simulink model "BMSModel"  (R2024b)
settings: Solver=VariableStepAuto  StartTime=0.0  StopTime=+inf  RelTol=1e-3
undefined symbols (not defined in the file): balancingRelaxationTime, balancingTime, ChargerParRes, ChargerRes, ChargingCurrentRequest, DeltaVTargetMin, DrivingCurrentRqst, InverterParRes, InverterRes, OpenCircuitInjection, PassiveBalancingRes, ShortCircuitInjection, sigbuilder_block, SystemState, SystemState_t
libraries: fl_lib x325, nesl_utility x223, batteryecm_lib x30, ee_lib x30
(part 1 of 33)
settings Model: SimulationMode=accelerator  Creator=E14  LastModifiedBy=E14

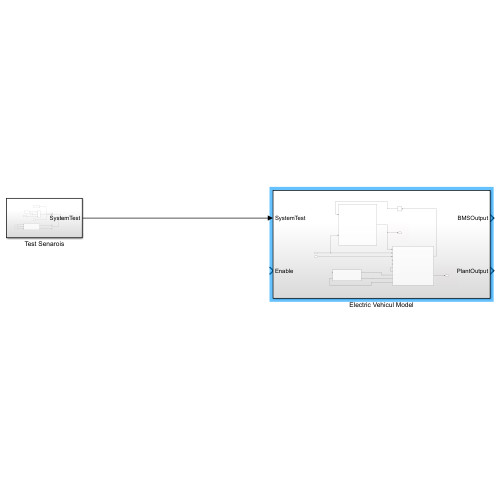
[thumbnail: Simulink's stored preview of the top-level diagram]
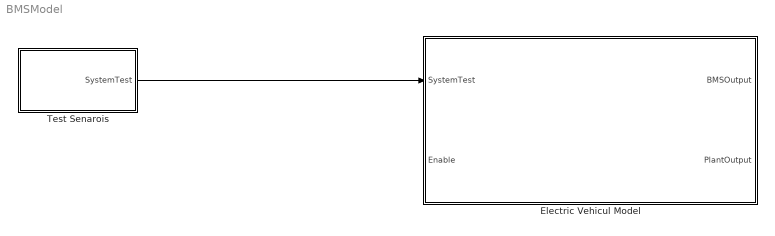
== system BMSModel ==
  Electric Vehicul Model : Subsystem
  Test Senarois : Subsystem
  wires:
  Test Senarois/1(SystemTest) -> Electric Vehicul Model/1(SystemTest)

== system BMSModel/Electric Vehicul Model ==
  SystemTest : Inport
  Enable : Inport  Port=2
  BMS model : Subsystem
  Contactors : Subsystem
  HV Battery : Subsystem
  Unit Delay : UnitDelay  InitialCondition=0.01  SampleTime=-1  HasFrameUpgradeWarning=on
  BMSOutput : Outport  OutDataTypeStr=Bus: BusOutputBMS
  PlantOutput : Outport  Port=2  OutDataTypeStr=Bus: BusSimulationOutput
  wires:
  BMS model/1(ToHVBattery) -> BMSOutput/1, HV Battery/1(DataFromBMS)
  Unit Delay/1 -> BMS model/1(DataFromHVBattery)
  HV Battery/1(ToBMS) -> Unit Delay/1
  SystemTest/1 -> HV Battery/2(SimulationTest), BMS model/2(SimulationTest)
  HV Battery/2(SimulationOutput) -> PlantOutput/1
  Enable/1 -> HV Battery/3(Enable)
  connections:
  HV Battery/LConn1 -- HV Battery/LConn2
  HV Battery/LConn5 -- Contactors/LConn1
  HV Battery/LConn3 -- Contactors/RConn1
  HV Battery/LConn4 -- Contactors/RConn2

== system BMSModel/Test Senarois ==
  Bus Creator : BusCreator  Inputs=SystemState,DrivingCurrentRqst,ChargingCurrentRequest  OutDataTypeStr=Bus: SimulationSystemInput
  Bus Creator1 : BusCreator  Inputs=ShortCircuitInjection,OpenCircuitInjection  OutDataTypeStr=Bus: SimulationBatteryPackInput
  Bus Creator2 : BusCreator  Inputs=2  OutDataTypeStr=Bus: BusSimulationInput  NonVirtualBus=on
  ChargingCurrentRqst : Constant  Value=42.56966044823757
  DrivingCurrentRqst : Constant  Value=30.1857592269321
  DrivingCurrentSelector : Constant
  MATLAB Function : MATLAB Function  TreatAsAtomicUnit=on
  ModelNumber : Constant  Value=3
  Signal Builder : Subsystem  Tag=STV Subsys  LoadFcn=sigbuilder_block('load');  StartFcn=sigbuilder_block('start');  StopFcn=sigbuilder_block('stop');  ClipboardFcn=sigbuilder_block('clipboard');  mask(Sigbuilder block){tuvar=[0:10]}
      mask help: Simulink:masks:SigbuilderBlock_MD
      mask init:
      | if ~strcmp(get_param(bdroot(gcbh),'SimulationStatus'),'stopped') tuvar = sigbuilder_block('maskInit'); end
      settings: CopyFcn=sigbuilder_block('copy');  DeleteFcn=sigbuilder_block('delete');  ModelCloseFcn=sigbuilder_block('modelClose');  PreSaveFcn=sigbuilder_block('preSave');  PauseFcn=sigbuilder_block('pause');  ContinueFcn=sigbuilder_block('continue');  NameChangeFcn=sigbuilder_block('namechange');  OpenFcn=sigbuilder_block('open',[5.4 5.4 1141.2 580.8 ]);  CloseFcn=sigbuilder_block('close');
      mask Display: plot(0, 0, 100, 100,[2, 2, 32, 32, 2], [68, 8, 8, 68, 68],[32, 2], [38, 38], [32, 19, 2],[53, 60, 44], [32, 17, 17, 2],[16, 16, 31, 31]);txt = getActiveGroup(gcbh);text(2, 100, txt,'verticalAlignment', 'top');
  Switch : Switch  Threshold=2  SaturateOnIntegerOverflow=off  InputSameDT=off
  SystemState : Constant  Value=SystemState_t(1)  OutDataTypeStr=Enum: SystemState_t
  cellNumber : Constant  Value=2
  faultType : Constant  Value=0
  SystemTest : Outport  OutDataTypeStr=Bus: BusSimulationInput
  wires:
  faultType/1 -> MATLAB Function/1(faultType)
  ChargingCurrentRqst/1 -> Bus Creator/3  "ChargingCurrentRequest"
  SystemState/1 -> Bus Creator/1  "SystemState"
  Bus Creator1/1 -> Bus Creator2/2  "SimulationBatteryPackInput"
  DrivingCurrentSelector/1 -> Switch/2
  Signal Builder/1(Current) -> Switch/1
  MATLAB Function/1(SCInjVector) -> Bus Creator1/1  "SCInjection"
  DrivingCurrentRqst/1 -> Switch/3
  Switch/1 -> Bus Creator/2  "DrivingCurrentRqst"
  MATLAB Function/2(OCInjVector) -> Bus Creator1/2  "OCInjection"
  Bus Creator/1 -> Bus Creator2/1  "SimulationSystemInput"
  cellNumber/1 -> MATLAB Function/3(cellNumber)
  ModelNumber/1 -> MATLAB Function/2(ModelNumber)
  Bus Creator2/1 -> SystemTest/1  "BusSimulationInput"

== system BMSModel/Electric Vehicul Model/BMS model ==
  DataFromHVBattery : Inport
  SimulationTest : Inport  Port=2
  Balancing system : Subsystem
  Bus Creator : BusCreator  Inputs=3
  Bus Selector : BusSelector  OutputSignals=BatteryPackData.ModelVoltageVector
  Bus Selector1 : BusSelector  OutputSignals=signal1
  Bus Selector2 : BusSelector  OutputSignals=BatteryPackData.ModelCurrentVector,BatteryPackData.ModelVoltageVector,BatteryPackData.ModelTempVector
  SOC_Estimation : Subsystem
  ToHVBattery : Outport
  wires:
  DataFromHVBattery/1 -> Bus Selector2/1, Bus Selector/1
  Bus Selector/1 -> Balancing system/2(SegmentVoltageVector)  "<ModelVoltageVector>"
  SimulationTest/1 -> Bus Selector1/1
  Bus Selector1/1 -> Balancing system/1(SystemState)  "<signal1>"
  Balancing system/1(balancingCmd) -> Bus Creator/1  "balancingCmd"
  Balancing system/2(balancingDeltaVCell) -> Bus Creator/2  "balancingDeltaVCell"
  Balancing system/3(balancingFlags) -> Bus Creator/3  "balancingFlags"
  Bus Creator/1 -> ToHVBattery/1  "Balancing"
  Bus Selector2/1 -> SOC_Estimation/1(In2)  "<ModelCurrentVector>"
  Bus Selector2/2 -> SOC_Estimation/2(In1)  "<ModelVoltageVector>"
  Bus Selector2/3 -> SOC_Estimation/3(In3)  "<ModelTempVector>"

== system BMSModel/Electric Vehicul Model/Contactors ==
  DichargerCmd : Inport
  Bat+ : PMIOPort  Side=Left
  Charger : PMIOPort  Port=2  Side=Right
  Inverter+ : PMIOPort  Port=3  Side=Right

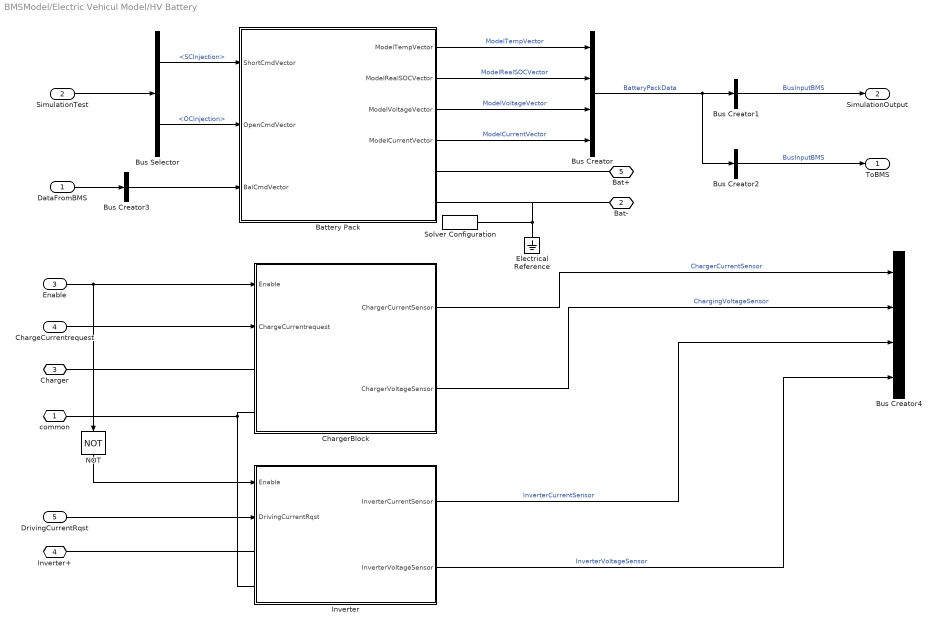
== system BMSModel/Electric Vehicul Model/HV Battery ==
  DataFromBMS : Inport
  SimulationTest : Inport  Port=2  OutDataTypeStr=Bus: BusSimulationInput
  Enable : Inport  Port=3
  ChargeCurrentrequest : Inport  Port=4
  DrivingCurrentRqst : Inport  Port=5
  Battery Pack : Subsystem
  Bus Creator : BusCreator  Inputs=4
  Bus Creator1 : BusCreator  Inputs=1
  Bus Creator2 : BusCreator  Inputs=1
  Bus Creator3 : BusCreator  Inputs=1
  Bus Creator4 : BusCreator  Inputs=4
  Bus Selector : BusSelector  OutputSignals=SimulationBatteryPackInput.SCInjection,SimulationBatteryPackInput.OCInjection
  ChargerBlock : Subsystem
  Electrical Reference : fl_lib/Electrical/Electrical Elements/Electrical Reference
  Inverter : Subsystem
  NOT : Logic  Operator=NOT  IconShape=distinctive  AllPortsSameDT=off  OutDataTypeStr=boolean
  Solver Configuration : nesl_utility/Solver Configuration  PhysicalDomain=network_engine_domain  SubClassName=solver  LeftPortType=input  RightPortType=generic  Tones=[]  Harmonics=[]  SimulateNoise=off  Profile=off  UseLocalSampling=off  EnableSwitchedLinearOptims=on  FrequencyDomain=off  UseCCode=off  RelTol=0.001  AbsTol=1e-06  MinStep=1e-09  ToWorkspace=off  RevertToSquareIcSolve=off  NumHomotopyIterations=100  PhasorMode=off  PhasorModeNumHarmonics=1  ConsistencySolver=NEWTON_FTOL  IndexReductionMethod=DerivativeReplacement  DoDC=off  ResidualTolerance=1e-09  ConsistencyTolSource=GLOBAL  ConsistencyAbsTol=0.001  ConsistencyRelTol=0.001  ConsistencyTolFactor=0.001  UseLocalSolver=off  LocalSolverChoice=NE_BACKWARD_EULER_ADVANCER  ResolveIndetEquations=on  FunctionEvalNumThread=1  LocalSolverSampleTime=0.001  DoFixedCost=off  MaxNonlinIter=3  MaxModeIter=2  ComputeImpulses=off  ImpulseIterations=2  LinearAlgebra=auto  LinearAlgebraNumThread=0  EquationFormulation=NE_TIME_EF  DelaysMemoryBudget=1024  AutomaticFiltering=on  FilteringTimeConstant=0.001  PartitionStorageMethod=AS_NEEDED  PartitionMemoryBudget=1024  PartitionMethod=ROBUST  MultibodyUseLocalSolver=off  MultibodyLocalSolverChoice=ODE1  MultibodyLocalSolverSampleTime=0.001
  common : PMIOPort  Side=Left
  Bat- : PMIOPort  Port=2  Side=Left
  Charger : PMIOPort  Port=3  Side=Left
  Inverter+ : PMIOPort  Port=4  Side=Left
  Bat+ : PMIOPort  Port=5  Side=Left
  ToBMS : Outport  OutDataTypeStr=Bus: BusSimulationOutput
  SimulationOutput : Outport  Port=2  OutDataTypeStr=Bus: BusSimulationOutput
  wires:
  Battery Pack/2(ModelRealSOCVector) -> Bus Creator/2  "ModelRealSOCVector"
  Bus Creator/1 -> Bus Creator2/1, Bus Creator1/1  "BatteryPackData"
  Battery Pack/1(ModelTempVector) -> Bus Creator/1  "ModelTempVector"
  Bus Creator1/1 -> SimulationOutput/1  "BusInputBMS"
  Bus Selector/2 -> Battery Pack/2(OpenCmdVector)  "<OCInjection>"
  Battery Pack/4(ModelCurrentVector) -> Bus Creator/4  "ModelCurrentVector"
  Bus Selector/1 -> Battery Pack/1(ShortCmdVector)  "<SCInjection>"
  Battery Pack/3(ModelVoltageVector) -> Bus Creator/3  "ModelVoltageVector"
  Bus Creator2/1 -> ToBMS/1  "BusInputBMS"
  SimulationTest/1 -> Bus Selector/1
  DataFromBMS/1 -> Bus Creator3/1
  Bus Creator3/1 -> Battery Pack/3(BalCmdVector)
  ChargerBlock/1(ChargerCurrentSensor) -> Bus Creator4/1  "ChargerCurrentSensor"
  ChargerBlock/2(ChargerVoltageSensor) -> Bus Creator4/2  "ChargingVoltageSensor"
  Inverter/1(InverterCurrentSensor) -> Bus Creator4/3  "InverterCurrentSensor"
  Inverter/2(InverterVoltageSensor) -> Bus Creator4/4  "InverterVoltageSensor"
  Enable/1 -> ChargerBlock/1(Enable), NOT/1
  NOT/1 -> Inverter/1(Enable)
  ChargeCurrentrequest/1 -> ChargerBlock/2(ChargeCurrentrequest)
  DrivingCurrentRqst/1 -> Inverter/2(DrivingCurrentRqst)
  connections:
  Solver Configuration/RConn1 -- Battery Pack/RConn2 -- Bat-/RConn1 -- Electrical Reference/LConn1
  Inverter/LConn2 -- common/RConn1 -- ChargerBlock/LConn2
  Charger/RConn1 -- ChargerBlock/LConn1
  Inverter+/RConn1 -- Inverter/LConn1
  Bat+/RConn1 -- Battery Pack/RConn1

== system BMSModel/Test Senarois/Signal Builder ==
  Demux : Demux  Tag=STV Demux  Outputs=1
      settings: DisplayOption=none
  FromWs : FromWorkspace  Tag=STV FromWs  VariableName=tuvar
      SigBuilderData=|
      | common.afterFinalStr = 'Extrapolate'
      | common.sampleTime = 0
      | dataSet.timeRange = [0 2474]
      | dataSet.name = 'FTP-75 Drive Cycle'
      | sbobj.Groups.Name = 'FTP-75 Drive Cycle'
      | sbobj.Groups.Signals.Name = 'Current'
      | sbobj.Groups.Signals.XData = [0 0.00166966283167 0.00489865696245 0.00887290141926 0.01 0.0132228027433 0.0164456054866 0.0196684082299 0.02 0.0216579588505 0.0216579588505 0.023878217756 ... (264079 elements, 1x264079)]
      | sbobj.Groups.Signals.YData = [0 0 0 0 0 0 0 0 0 0 -7.47487403974e-31 -1.06202348499e-17 ... (264079 elements, 1x264079)]
      | sbobj.Groups.TimeRange = [0 2474]
  Current : Outport  Tag=STV Outport
  wires:
  FromWs/1 -> Demux/1
  Demux/1 -> Current/1

== system BMSModel/Electric Vehicul Model/BMS model/Balancing system ==
  SystemState : Inport  OutDataTypeStr=Enum: SystemState_t
  SegmentVoltageVector : Inport  Port=2
  Chart1 : Stateflow chart  TreatAsAtomicUnit=on
      settings: PortSchema="{\"entries\":[{\"content\":{\"sides\":[{\"content\":{\"connectorIds\":[\"In1\",\"In2\",\"In3\",\"In4\"]},\"type\":\"ConnectorPlacement.EquallySpacedRectSide\",\"uuid\":\"a2741edf-0a6a-4cdc-8864-68b68dbb5e7b\"},{\"content\":{\"connectorIds\":[\"Out2\",\"Out1\",\"Out3\"],\"side\":\"RIGHT\"},\"type\":\"ConnectorPlacement.EquallySpacedRectSide\",\"uuid\":\"3e33edf4-8d09-490e-8b71-f55d7866ff69\"},{\"content\":{\"connectorIds\":[],\"side\":\"TOP\"},\"type\":\"ConnectorPlacement.EquallySpacedRectSide\",\"uuid\":\"52eb0d7a-a4df-4df7-91e4-464aa4e0ab31\"}]},\"type\":\"ConnectorPlacement.EquallySpacedConnector\",\"uuid\":\"8927709b-3b25-412c-93a6-8f62d2d50716\"}],\"packageUris\":[\"http://schema.mathworks.com/mf0/Connector/R2020b\"],\"version\":\"1.0\"}"
  Chart2 : Stateflow chart  TreatAsAtomicUnit=on
      settings: PortSchema="{\"entries\":[{\"content\":{\"sides\":[{\"content\":{\"connectorIds\":[\"In1\",\"In2\",\"In3\",\"In4\"]},\"type\":\"ConnectorPlacement.EquallySpacedRectSide\",\"uuid\":\"a2741edf-0a6a-4cdc-8864-68b68dbb5e7b\"},{\"content\":{\"connectorIds\":[\"Out2\",\"Out1\",\"Out3\"],\"side\":\"RIGHT\"},\"type\":\"ConnectorPlacement.EquallySpacedRectSide\",\"uuid\":\"3e33edf4-8d09-490e-8b71-f55d7866ff69\"},{\"content\":{\"connectorIds\":[],\"side\":\"TOP\"},\"type\":\"ConnectorPlacement.EquallySpacedRectSide\",\"uuid\":\"52eb0d7a-a4df-4df7-91e4-464aa4e0ab31\"}]},\"type\":\"ConnectorPlacement.EquallySpacedConnector\",\"uuid\":\"8927709b-3b25-412c-93a6-8f62d2d50716\"}],\"packageUris\":[\"http://schema.mathworks.com/mf0/Connector/R2020b\"],\"version\":\"1.0\"}"
  Chart3 : Stateflow chart  TreatAsAtomicUnit=on
      settings: PortSchema="{\"entries\":[{\"content\":{\"sides\":[{\"content\":{\"connectorIds\":[\"In1\",\"In2\",\"In3\",\"In4\"]},\"type\":\"ConnectorPlacement.EquallySpacedRectSide\",\"uuid\":\"a2741edf-0a6a-4cdc-8864-68b68dbb5e7b\"},{\"content\":{\"connectorIds\":[\"Out2\",\"Out1\",\"Out3\"],\"side\":\"RIGHT\"},\"type\":\"ConnectorPlacement.EquallySpacedRectSide\",\"uuid\":\"3e33edf4-8d09-490e-8b71-f55d7866ff69\"},{\"content\":{\"connectorIds\":[],\"side\":\"TOP\"},\"type\":\"ConnectorPlacement.EquallySpacedRectSide\",\"uuid\":\"52eb0d7a-a4df-4df7-91e4-464aa4e0ab31\"}]},\"type\":\"ConnectorPlacement.EquallySpacedConnector\",\"uuid\":\"8927709b-3b25-412c-93a6-8f62d2d50716\"}],\"packageUris\":[\"http://schema.mathworks.com/mf0/Connector/R2020b\"],\"version\":\"1.0\"}"
  Matrix Concatenate : Concatenate  NumInputs=3  Mode=Multidimensional array  ConcatenateDimension=2
  Max : MinMax  Function=max
  Max1 : MinMax  Function=max
  Max2 : MinMax  Function=max
  Min : MinMax
  Min1 : MinMax
  Min2 : MinMax
  Min3 : MinMax  Function=max  Inputs=3
  Min4 : MinMax  Inputs=3
  Selector : Selector  NumberOfDimensions=2  InputPortWidth=3  IndexOptions=Starting index (dialog),Select all  Indices=1,1  OutputSizes=1,1
  Selector1 : Selector  NumberOfDimensions=2  InputPortWidth=3  IndexOptions=Starting index (dialog),Select all  Indices=2,1  OutputSizes=1,1
  Selector2 : Selector  NumberOfDimensions=2  InputPortWidth=3  IndexOptions=Starting index (dialog),Select all  Indices=3,1  OutputSizes=1,1
  Transpose : Math  Operator=transpose
  Vector Concatenate : Concatenate  NumInputs=3
  Vector Concatenate1 : Concatenate  NumInputs=3
  balancingCmd : Outport
  balancingDeltaVCell : Outport  Port=2
  balancingFlags : Outport  Port=3
  wires:
  Selector2/1 -> Max2/1, Min2/1, Chart3/2(SegmentVoltageVector)
  Transpose/1 -> balancingCmd/1
  Selector/1 -> Max/1, Min/1, Chart1/2(SegmentVoltageVector)
  Min3/1 -> Chart2/3(CellMaxV), Chart1/3(CellMaxV), Chart3/3(CellMaxV)
  Min/1 -> Min4/1
  SystemState/1 -> Chart2/1(SystemState), Chart3/1(SystemState), Chart1/1(SystemState)
  Min4/1 -> Chart2/4(CellMinV), Chart1/4(CellMinV), Chart3/4(CellMinV)
  SegmentVoltageVector/1 -> Selector/1, Selector1/1, Selector2/1
  Chart1/2(DeltaVCell) -> Vector Concatenate/1
  Min2/1 -> Min4/3
  Chart1/1(balancingCmd) -> Matrix Concatenate/1
  Chart1/3(balancingFlag) -> Vector Concatenate1/1
  Selector1/1 -> Min1/1, Max1/1, Chart2/2(SegmentVoltageVector)
  Matrix Concatenate/1 -> Transpose/1
  Chart3/1(balancingCmd) -> Matrix Concatenate/3
  Max2/1 -> Min3/3
  Chart2/1(balancingCmd) -> Matrix Concatenate/2
  Chart2/2(DeltaVCell) -> Vector Concatenate/2
  Max/1 -> Min3/1
  Chart3/2(DeltaVCell) -> Vector Concatenate/3
  Chart2/3(balancingFlag) -> Vector Concatenate1/2
  Chart3/3(balancingFlag) -> Vector Concatenate1/3
  Vector Concatenate1/1 -> balancingFlags/1
  Min1/1 -> Min4/2
  Vector Concatenate/1 -> balancingDeltaVCell/1
  Max1/1 -> Min3/2

== system BMSModel/Electric Vehicul Model/BMS model/SOC_Estimation ==
  In2 : Inport
  In1 : Inport  Port=2
  In3 : Inport  Port=3
  Out1 : Outport

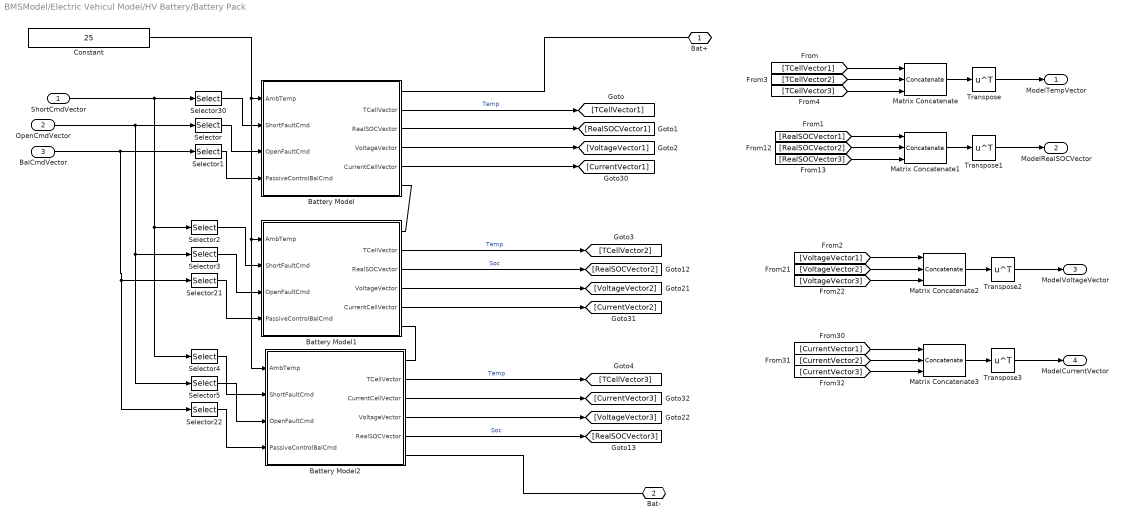
== system BMSModel/Electric Vehicul Model/HV Battery/Battery Pack ==
  ShortCmdVector : Inport
  OpenCmdVector : Inport  Port=2
  BalCmdVector : Inport  Port=3
  Battery Model : Subsystem
      settings: PortSchema="{\"entries\":[{\"content\":{\"sides\":[{\"content\":{\"connectorIds\":[\"In1\",\"In2\",\"In3\",\"In4\"]},\"type\":\"ConnectorPlacement.EquallySpacedRectSide\",\"uuid\":\"339fc2dc-73de-410a-a97e-1fe159b417b4\"},{\"content\":{\"connectorIds\":[\"RConn1\",\"Out1\",\"Out2\",\"Out3\",\"Out4\",\"RConn2\"],\"side\":\"RIGHT\"},\"type\":\"ConnectorPlacement.EquallySpacedRectSide\",\"uuid\":\"1ff17677-7e7c-48aa-9b47-364d19db4b22\"},{\"content\":{\"side\":\"TOP\"},\"type\":\"ConnectorPlacement.EquallySpacedRectSide\",\"uuid\":\"a6a1d56b-2099-4034-99b1-512debfe3694\"}]},\"type\":\"ConnectorPlacement.EquallySpacedConnector\",\"uuid\":\"fb04511b-02af-41ab-983c-5fc3ec8a5a5f\"}],\"packageUris\":[\"http://schema.mathworks.com/mf0/Connector/R2020b\"],\"version\":\"1.0\"}"
  Battery Model1 : Subsystem
      settings: PortSchema="{\"entries\":[{\"content\":{\"sides\":[{\"content\":{\"connectorIds\":[\"In1\",\"In2\",\"In3\",\"In4\"]},\"type\":\"ConnectorPlacement.EquallySpacedRectSide\",\"uuid\":\"339fc2dc-73de-410a-a97e-1fe159b417b4\"},{\"content\":{\"connectorIds\":[\"RConn1\",\"Out1\",\"Out2\",\"Out3\",\"Out4\",\"RConn2\"],\"side\":\"RIGHT\"},\"type\":\"ConnectorPlacement.EquallySpacedRectSide\",\"uuid\":\"1ff17677-7e7c-48aa-9b47-364d19db4b22\"},{\"content\":{\"side\":\"TOP\"},\"type\":\"ConnectorPlacement.EquallySpacedRectSide\",\"uuid\":\"a6a1d56b-2099-4034-99b1-512debfe3694\"}]},\"type\":\"ConnectorPlacement.EquallySpacedConnector\",\"uuid\":\"fb04511b-02af-41ab-983c-5fc3ec8a5a5f\"}],\"packageUris\":[\"http://schema.mathworks.com/mf0/Connector/R2020b\"],\"version\":\"1.0\"}"
  Battery Model2 : Subsystem
      settings: PortSchema="{\"entries\":[{\"content\":{\"sides\":[{\"content\":{\"connectorIds\":[\"In1\",\"In2\",\"In3\",\"In4\"]},\"type\":\"ConnectorPlacement.EquallySpacedRectSide\",\"uuid\":\"339fc2dc-73de-410a-a97e-1fe159b417b4\"},{\"content\":{\"connectorIds\":[\"RConn1\",\"Out1\",\"Out2\",\"Out3\",\"Out4\",\"RConn2\"],\"side\":\"RIGHT\"},\"type\":\"ConnectorPlacement.EquallySpacedRectSide\",\"uuid\":\"1ff17677-7e7c-48aa-9b47-364d19db4b22\"},{\"content\":{\"side\":\"TOP\"},\"type\":\"ConnectorPlacement.EquallySpacedRectSide\",\"uuid\":\"a6a1d56b-2099-4034-99b1-512debfe3694\"}]},\"type\":\"ConnectorPlacement.EquallySpacedConnector\",\"uuid\":\"fb04511b-02af-41ab-983c-5fc3ec8a5a5f\"}],\"packageUris\":[\"http://schema.mathworks.com/mf0/Connector/R2020b\"],\"version\":\"1.0\"}"
  Constant : Constant  Value=25
  Matrix Concatenate{,1..3} (4 alike) : Concatenate  NumInputs=3  Mode=Multidimensional array  ConcatenateDimension=2
  Selector : Selector  NumberOfDimensions=2  InputPortWidth=10  IndexOptions=Starting index (dialog),Select all  Indices=1,1  OutputSizes=1,1
  Selector1 : Selector  NumberOfDimensions=2  InputPortWidth=10  IndexOptions=Starting index (dialog),Select all  Indices=1,1  OutputSizes=1,1
  Selector2 : Selector  NumberOfDimensions=2  InputPortWidth=10  IndexOptions=Starting index (dialog),Select all  Indices=2,1  OutputSizes=1,1
  Selector21 : Selector  NumberOfDimensions=2  InputPortWidth=10  IndexOptions=Starting index (dialog),Select all  Indices=2,1  OutputSizes=1,1
  Selector22 : Selector  NumberOfDimensions=2  InputPortWidth=10  IndexOptions=Starting index (dialog),Select all  Indices=3,1  OutputSizes=1,1
  Selector3 : Selector  NumberOfDimensions=2  InputPortWidth=10  IndexOptions=Starting index (dialog),Select all  Indices=2,1  OutputSizes=1,1
  Selector30 : Selector  NumberOfDimensions=2  InputPortWidth=10  IndexOptions=Starting index (dialog),Select all  Indices=1,1  OutputSizes=1,1
  Selector4 : Selector  NumberOfDimensions=2  InputPortWidth=10  IndexOptions=Starting index (dialog),Select all  Indices=3,1  OutputSizes=1,1
  Selector5 : Selector  NumberOfDimensions=2  InputPortWidth=10  IndexOptions=Starting index (dialog),Select all  Indices=3,1  OutputSizes=1,1
  Transpose{,1..3} (4 alike) : Math  Operator=transpose
  Bat+ : PMIOPort  Side=Right
  Bat- : PMIOPort  Port=2  Side=Right
  ModelTempVector : Outport
  ModelRealSOCVector : Outport  Port=2
  ModelVoltageVector : Outport  Port=3
  ModelCurrentVector : Outport  Port=4
  wires:
  ShortCmdVector/1 -> Selector4/1, Selector2/1, Selector30/1
  Battery Model/1(TCellVector) -> [TCellVector1]  "Temp"
  Selector21/1 -> Battery Model1/4(PassiveControlBalCmd)
  OpenCmdVector/1 -> Selector5/1, Selector3/1, Selector/1
  Battery Model1/1(TCellVector) -> [TCellVector2]  "Temp"
  BalCmdVector/1 -> Selector22/1, Selector21/1, Selector1/1
  Battery Model1/3(VoltageVector) -> [VoltageVector2]
  Selector2/1 -> Battery Model1/2(ShortFaultCmd)
  Transpose2/1 -> ModelVoltageVector/1
  Matrix Concatenate1/1 -> Transpose1/1
  Matrix Concatenate3/1 -> Transpose3/1
  Battery Model2/2(CurrentCellVector) -> [CurrentVector3]
  Battery Model2/3(VoltageVector) -> [VoltageVector3]
  [TCellVector3] -> Matrix Concatenate/3
  [VoltageVector2] -> Matrix Concatenate2/2
  [TCellVector1] -> Matrix Concatenate/1
  Transpose3/1 -> ModelCurrentVector/1
  Constant/1 -> Battery Model2/1(AmbTemp), Battery Model1/1(AmbTemp), Battery Model/1(AmbTemp)
  Selector30/1 -> Battery Model/2(ShortFaultCmd)
  Battery Model2/1(TCellVector) -> [TCellVector3]  "Temp"
  [CurrentVector2] -> Matrix Concatenate3/2
  Selector3/1 -> Battery Model1/3(OpenFaultCmd)
  [RealSOCVector2] -> Matrix Concatenate1/2
  [CurrentVector3] -> Matrix Concatenate3/3
  Transpose1/1 -> ModelRealSOCVector/1
  [CurrentVector1] -> Matrix Concatenate3/1
  Matrix Concatenate2/1 -> Transpose2/1
  Transpose/1 -> ModelTempVector/1
  [RealSOCVector1] -> Matrix Concatenate1/1
  Matrix Concatenate/1 -> Transpose/1
  Selector5/1 -> Battery Model2/3(OpenFaultCmd)
  Selector/1 -> Battery Model/3(OpenFaultCmd)
  [RealSOCVector3] -> Matrix Concatenate1/3
  [TCellVector2] -> Matrix Concatenate/2
  Battery Model2/4(RealSOCVector) -> [RealSOCVector3]  "Soc"
  Battery Model1/2(RealSOCVector) -> [RealSOCVector2]  "Soc"
  [VoltageVector1] -> Matrix Concatenate2/1
  [VoltageVector3] -> Matrix Concatenate2/3
  Battery Model/2(RealSOCVector) -> [RealSOCVector1]
  Selector22/1 -> Battery Model2/4(PassiveControlBalCmd)
  Selector1/1 -> Battery Model/4(PassiveControlBalCmd)
  Battery Model/3(VoltageVector) -> [VoltageVector1]
  Battery Model/4(CurrentCellVector) -> [CurrentVector1]
  Selector4/1 -> Battery Model2/2(ShortFaultCmd)
  Battery Model1/4(CurrentCellVector) -> [CurrentVector2]
  connections:
  Battery Model1/RConn2 -- Battery Model2/RConn1
  Battery Model/RConn2 -- Battery Model1/RConn1
  Battery Model2/RConn2 -- Bat-/RConn1
  Battery Model/RConn1 -- Bat+/RConn1

== system BMSModel/Electric Vehicul Model/HV Battery/ChargerBlock ==
  Enable : Inport
  ChargeCurrentrequest : Inport  Port=2
  Cast To Double : DataTypeConversion  OutDataTypeStr=double  SaturateOnIntegerOverflow=off  RndMeth=Floor
  Cast To Double1 : DataTypeConversion  OutDataTypeStr=double  SaturateOnIntegerOverflow=off  RndMeth=Floor
  Cast To Double2 : DataTypeConversion  OutDataTypeStr=double  SaturateOnIntegerOverflow=off  RndMeth=Floor
  ChargerCap : fl_lib/Electrical/Electrical Elements/Capacitor  c=ChargerCap F  r=1e-6 Ohm  g=0 1/Ohm  i_specify=off  i_priority=None  i=0 A  i_nominal_specify=off  i_nominal_value=1  i_nominal_unit=A  v_specify=off  v_priority=None  v=0 V  v_nominal_specify=off  v_nominal_value=1  v_nominal_unit=V  vc_specify=off  vc_priority=High  vc=0 V  vc_nominal_specify=off  vc_nominal_value=1  vc_nominal_unit=V
  ChargerParResistance : fl_lib/Electrical/Electrical Elements/Resistor  R=ChargerParRes Ohm  i_specify=off  i_priority=None  i=0 A  i_nominal_specify=off  i_nominal_value=1  i_nominal_unit=A  v_specify=off  v_priority=None  v=0 V  v_nominal_specify=off  v_nominal_value=1  v_nominal_unit=V
  ChargerResistance : fl_lib/Electrical/Electrical Elements/Resistor  R=ChargerRes Ohm  i_specify=off  i_priority=None  i=0 A  i_nominal_specify=off  i_nominal_value=1  i_nominal_unit=A  v_specify=off  v_priority=None  v=0 V  v_nominal_specify=off  v_nominal_value=1  v_nominal_unit=V
  Constant3 : Constant  Value=0
  Controlled Current Source1 : fl_lib/Electrical/Electrical Sources/Controlled Current Source
  Current Sensor1 : fl_lib/Electrical/Electrical Sensors/Current Sensor
  PS-Simulink Converter : nesl_utility/PS-Simulink Converter  PhysicalDomain=network_engine_domain  SubClassName=ps_output  LeftPortType=input  RightPortType=output  PseudoPeriodic=off  Frequencies=[]  VectorFormat=1-D array  Unit=inherit  AffineConversion=off
  PS-Simulink Converter1 : nesl_utility/PS-Simulink Converter  PhysicalDomain=network_engine_domain  SubClassName=ps_output  LeftPortType=input  RightPortType=output  PseudoPeriodic=off  Frequencies=[]  VectorFormat=1-D array  Unit=inherit  AffineConversion=off
  Simulink-PS Converter1 : nesl_utility/Simulink-PS Converter  PhysicalDomain=network_engine_domain  SubClassName=ps_input  LeftPortType=input  RightPortType=output  PseudoPeriodic=off  Frequencies=[]  NoiseDistribution=none  NoiseParameters=[]  Unit=1  AffineConversion=off  FilteringAndDerivatives=provide  SimscapeFilterOrder=1  InputFilterTimeConstant=0.001  UdotUserProvided=0
  Switch1 : Switch  Criteria=u2 > Threshold  SaturateOnIntegerOverflow=off  InputSameDT=off
  Voltage Sensor1 : fl_lib/Electrical/Electrical Sensors/Voltage Sensor
  Charger+ : PMIOPort  Side=Left
  Charger- : PMIOPort  Port=2  Side=Left
  ChargerCurrentSensor : Outport
  ChargerVoltageSensor : Outport  Port=2
  wires:
  Cast To Double/1 -> Simulink-PS Converter1/1
  Constant3/1 -> Switch1/3
  PS-Simulink Converter/1 -> Cast To Double1/1
  PS-Simulink Converter1/1 -> Cast To Double2/1
  Cast To Double1/1 -> ChargerCurrentSensor/1
  Cast To Double2/1 -> ChargerVoltageSensor/1
  Enable/1 -> Switch1/2
  ChargeCurrentrequest/1 -> Switch1/1
  Switch1/1 -> Cast To Double/1
  connections:
  Controlled Current Source1/LConn1 -- Current Sensor1/LConn1
  Voltage Sensor1/LConn1 -- ChargerResistance/RConn1 -- Current Sensor1/RConn2
  Simulink-PS Converter1/RConn1 -- Controlled Current Source1/RConn1
  ChargerCap/LConn1 -- Charger+/RConn1 -- ChargerParResistance/LConn1 -- ChargerResistance/LConn1
  PS-Simulink Converter1/LConn1 -- Voltage Sensor1/RConn1
  Controlled Current Source1/RConn2 -- Charger-/RConn1 -- ChargerCap/RConn1 -- ChargerParResistance/RConn1 -- Voltage Sensor1/RConn2
  Current Sensor1/RConn1 -- PS-Simulink Converter/LConn1

== system BMSModel/Electric Vehicul Model/HV Battery/Inverter ==
  Enable : Inport
  DrivingCurrentRqst : Inport  Port=2
  Cast To Double : DataTypeConversion  OutDataTypeStr=double  SaturateOnIntegerOverflow=off  RndMeth=Floor
  Cast To Double1 : DataTypeConversion  OutDataTypeStr=double  SaturateOnIntegerOverflow=off  RndMeth=Floor
  Cast To Double2 : DataTypeConversion  OutDataTypeStr=double  SaturateOnIntegerOverflow=off  RndMeth=Floor
  Constant3 : Constant  Value=0
  Controlled Current Source1 : fl_lib/Electrical/Electrical Sources/Controlled Current Source
  Current Sensor1 : fl_lib/Electrical/Electrical Sensors/Current Sensor
  InverterCap : fl_lib/Electrical/Electrical Elements/Capacitor  c=InverterCap F  r=1e-6 Ohm  g=0 1/Ohm  i_specify=off  i_priority=None  i=0 A  i_nominal_specify=off  i_nominal_value=1  i_nominal_unit=A  v_specify=off  v_priority=None  v=0 V  v_nominal_specify=off  v_nominal_value=1  v_nominal_unit=V  vc_specify=off  vc_priority=High  vc=0 V  vc_nominal_specify=off  vc_nominal_value=1  vc_nominal_unit=V
  InverterParResistance : fl_lib/Electrical/Electrical Elements/Resistor  R=InverterParRes Ohm  i_specify=off  i_priority=None  i=0 A  i_nominal_specify=off  i_nominal_value=1  i_nominal_unit=A  v_specify=off  v_priority=None  v=0 V  v_nominal_specify=off  v_nominal_value=1  v_nominal_unit=V
  InverterResistance : fl_lib/Electrical/Electrical Elements/Resistor  R=InverterRes Ohm  i_specify=off  i_priority=None  i=0 A  i_nominal_specify=off  i_nominal_value=1  i_nominal_unit=A  v_specify=off  v_priority=None  v=0 V  v_nominal_specify=off  v_nominal_value=1  v_nominal_unit=V
  PS-Simulink Converter : nesl_utility/PS-Simulink Converter  PhysicalDomain=network_engine_domain  SubClassName=ps_output  LeftPortType=input  RightPortType=output  PseudoPeriodic=off  Frequencies=[]  VectorFormat=1-D array  Unit=inherit  AffineConversion=off
  PS-Simulink Converter1 : nesl_utility/PS-Simulink Converter  PhysicalDomain=network_engine_domain  SubClassName=ps_output  LeftPortType=input  RightPortType=output  PseudoPeriodic=off  Frequencies=[]  VectorFormat=1-D array  Unit=inherit  AffineConversion=off
  Simulink-PS Converter1 : nesl_utility/Simulink-PS Converter  PhysicalDomain=network_engine_domain  SubClassName=ps_input  LeftPortType=input  RightPortType=output  PseudoPeriodic=off  Frequencies=[]  NoiseDistribution=none  NoiseParameters=[]  Unit=1  AffineConversion=off  FilteringAndDerivatives=provide  SimscapeFilterOrder=1  InputFilterTimeConstant=0.001  UdotUserProvided=0
  Switch1 : Switch  Criteria=u2 > Threshold  SaturateOnIntegerOverflow=off  InputSameDT=off
  Voltage Sensor1 : fl_lib/Electrical/Electrical Sensors/Voltage Sensor
  Inverter+ : PMIOPort  Side=Left
  Inverter- : PMIOPort  Port=2  Side=Left
  InverterCurrentSensor : Outport
  InverterVoltageSensor : Outport  Port=2
  wires:
  Cast To Double/1 -> Simulink-PS Converter1/1
  Constant3/1 -> Switch1/3
  PS-Simulink Converter/1 -> Cast To Double1/1
  PS-Simulink Converter1/1 -> Cast To Double2/1
  Switch1/1 -> Cast To Double/1
  DrivingCurrentRqst/1 -> Switch1/1
  Cast To Double1/1 -> InverterCurrentSensor/1
  Cast To Double2/1 -> InverterVoltageSensor/1
  Enable/1 -> Switch1/2
  connections:
  Controlled Current Source1/RConn2 -- Current Sensor1/LConn1
  Voltage Sensor1/RConn2 -- InverterParResistance/RConn1 -- Inverter-/RConn1 -- InverterCap/RConn1 -- Controlled Current Source1/LConn1
  Simulink-PS Converter1/RConn1 -- Controlled Current Source1/RConn1
  InverterCap/LConn1 -- Inverter+/RConn1 -- InverterParResistance/LConn1 -- InverterResistance/LConn1
  InverterResistance/RConn1 -- Voltage Sensor1/LConn1 -- Current Sensor1/RConn2
  PS-Simulink Converter/LConn1 -- Current Sensor1/RConn1
  PS-Simulink Converter1/LConn1 -- Voltage Sensor1/RConn1

== system BMSModel/Electric Vehicul Model/HV Battery/Battery Pack/Battery Model ==
  AmbTemp : Inport
  ShortFaultCmd : Inport  Port=2
  OpenFaultCmd : Inport  Port=3
  PassiveControlBalCmd : Inport  Port=4
  Battery_Model : Subsystem
  Subsystem1 : Subsystem
  BattMod+ : PMIOPort  Side=Right
  BattMod- : PMIOPort  Port=2  Side=Right
  TCellVector : Outport
  RealSOCVector : Outport  Port=2
  VoltageVector : Outport  Port=3
  CurrentCellVector : Outport  Port=4
  wires:
  OpenFaultCmd/1 -> Battery_Model/3(OpenFaultCmd)
  Battery_Model/1(TCellVector) -> TCellVector/1
  Subsystem1/1(VoltageVector) -> VoltageVector/1
  Battery_Model/2(RealSocVector) -> RealSOCVector/1
  Battery_Model/3(CurrentCellVector) -> CurrentCellVector/1
  AmbTemp/1 -> Battery_Model/1(AmbTemp)
  PassiveControlBalCmd/1 -> Subsystem1/1(PassiveControlBalCmd1)
  ShortFaultCmd/1 -> Battery_Model/2(ShortFaultCmd)
  connections:
  Subsystem1/LConn7 -- Battery_Model/RConn7
  Subsystem1/LConn1 -- BattMod+/RConn1 -- Battery_Model/RConn1
  Subsystem1/LConn11 -- BattMod-/RConn1 -- Battery_Model/RConn11
  Subsystem1/LConn9 -- Battery_Model/RConn9
  Subsystem1/LConn6 -- Battery_Model/RConn6
  Subsystem1/LConn10 -- Battery_Model/RConn10
  Subsystem1/LConn4 -- Battery_Model/RConn4
  Subsystem1/LConn5 -- Battery_Model/RConn5
  Subsystem1/LConn8 -- Battery_Model/RConn8
  Subsystem1/LConn3 -- Battery_Model/RConn3
  Subsystem1/LConn2 -- Battery_Model/RConn2

== system BMSModel/Electric Vehicul Model/HV Battery/Battery Pack/Battery Model1 ==
  AmbTemp : Inport
  ShortFaultCmd : Inport  Port=2
  OpenFaultCmd : Inport  Port=3
  PassiveControlBalCmd : Inport  Port=4
  Battery_Model : Subsystem
  Subsystem1 : Subsystem
  BattMod+ : PMIOPort  Side=Right
  BattMod- : PMIOPort  Port=2  Side=Right
  TCellVector : Outport
  RealSOCVector : Outport  Port=2
  VoltageVector : Outport  Port=3
  CurrentCellVector : Outport  Port=4
  wires:
  ShortFaultCmd/1 -> Battery_Model/2(ShortFaultCmd)
  PassiveControlBalCmd/1 -> Subsystem1/1(PassiveControlBalCmd1)
  AmbTemp/1 -> Battery_Model/1(AmbTemp)
  Battery_Model/3(CurrentCellVector) -> CurrentCellVector/1
  Battery_Model/2(RealSocVector) -> RealSOCVector/1
  Subsystem1/1(VoltageVector) -> VoltageVector/1
  Battery_Model/1(TCellVector) -> TCellVector/1
  OpenFaultCmd/1 -> Battery_Model/3(OpenFaultCmd)
  connections:
  Subsystem1/LConn2 -- Battery_Model/RConn2
  Subsystem1/LConn3 -- Battery_Model/RConn3
  Subsystem1/LConn8 -- Battery_Model/RConn8
  Subsystem1/LConn5 -- Battery_Model/RConn5
  Subsystem1/LConn4 -- Battery_Model/RConn4
  Subsystem1/LConn10 -- Battery_Model/RConn10
  Subsystem1/LConn6 -- Battery_Model/RConn6
  Subsystem1/LConn9 -- Battery_Model/RConn9
  BattMod-/RConn1 -- Subsystem1/LConn11 -- Battery_Model/RConn11
  BattMod+/RConn1 -- Subsystem1/LConn1 -- Battery_Model/RConn1
  Subsystem1/LConn7 -- Battery_Model/RConn7

== system BMSModel/Electric Vehicul Model/HV Battery/Battery Pack/Battery Model2 ==
  AmbTemp : Inport
  ShortFaultCmd : Inport  Port=2
  OpenFaultCmd : Inport  Port=3
  PassiveControlBalCmd : Inport  Port=4
  Battery_Model : Subsystem
  Subsystem1 : Subsystem
  BattMod+ : PMIOPort  Side=Right
  BattMod- : PMIOPort  Port=2  Side=Right
  TCellVector : Outport
  CurrentCellVector : Outport  Port=2
  VoltageVector : Outport  Port=3
  RealSOCVector : Outport  Port=4
  wires:
  OpenFaultCmd/1 -> Battery_Model/3(OpenFaultCmd)
  Battery_Model/1(TCellVector) -> TCellVector/1
  Subsystem1/1(VoltageVector) -> VoltageVector/1
  Battery_Model/2(RealSocVector) -> RealSOCVector/1
  Battery_Model/3(CurrentCellVector) -> CurrentCellVector/1
  AmbTemp/1 -> Battery_Model/1(AmbTemp)
  PassiveControlBalCmd/1 -> Subsystem1/1(PassiveControlBalCmd1)
  ShortFaultCmd/1 -> Battery_Model/2(ShortFaultCmd)
  connections:
  Subsystem1/LConn7 -- Battery_Model/RConn7
  Battery_Model/RConn1 -- Subsystem1/LConn1 -- BattMod+/RConn1
  Battery_Model/RConn11 -- Subsystem1/LConn11 -- BattMod-/RConn1
  Subsystem1/LConn9 -- Battery_Model/RConn9
  Subsystem1/LConn6 -- Battery_Model/RConn6
  Subsystem1/LConn10 -- Battery_Model/RConn10
  Subsystem1/LConn4 -- Battery_Model/RConn4
  Subsystem1/LConn5 -- Battery_Model/RConn5
  Subsystem1/LConn8 -- Battery_Model/RConn8
  Subsystem1/LConn3 -- Battery_Model/RConn3
  Subsystem1/LConn2 -- Battery_Model/RConn2
